simulink model "Simulatore_COMAU_UBHAND_IV_manipolazione_interna"  (Simulink 7.6)
settings: Solver=ode45  StartTime=0.0  StopTime=inf  RelTol=1e-3
values from neighbouring files (symbols this model uses):
  a_f = zeros(4,5)    [KinematicData.m]
  alpha_f = zeros(4,5)    [KinematicData.m]
  d_f = zeros(4,5)    [KinematicData.m]
  dc4_1 = 660    [KinematicData.m]
  T6HAND = [1 0 0 0;0 1 0 0; 0 0 1 357.48+95; 0 0 0 1]    [KinematicData.m]
  teta_d = atan(647.07/130)    [KinematicData.m]
  teta_f = zeros(4,5)    [KinematicData.m]
undefined symbols (not defined in the file or in its folder): a_c, a_w, alpha_c, alpha_w, d_c, d_w, Dt_fingers, teta_c
libraries: simulink x281, dspmtrx3 x171, aerolibutil x163, aerolibconvert2 x64, simulink_extras x46, vrlib x26, aerolibtransform2 x7, dspnetwork x1
(part 3 of 15)

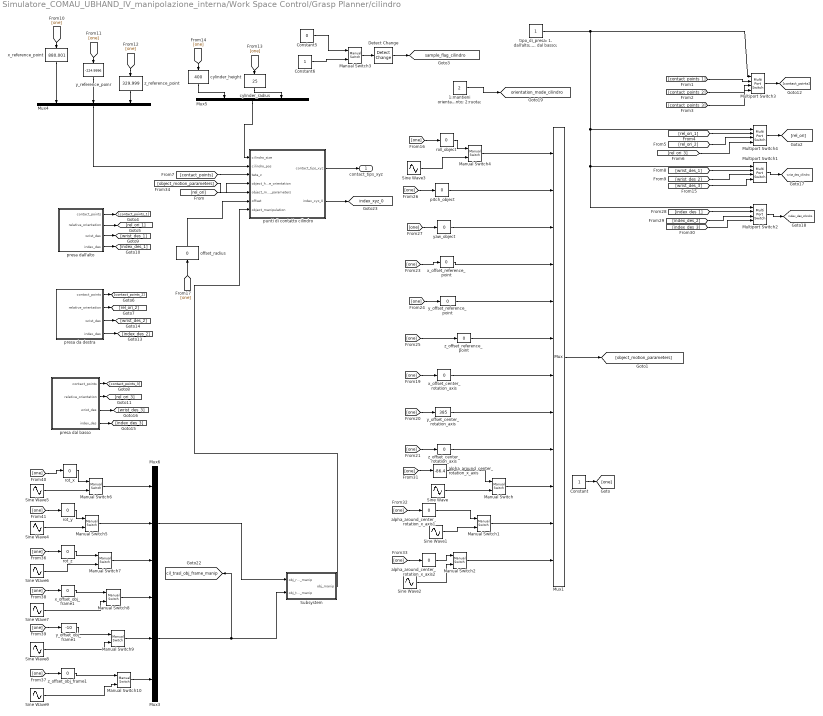
== system Simulatore_COMAU_UBHAND_IV_manipolazione_interna/Work Space Control/Grasp Planner/cilindro ==
  1:mantieni orientamento; 2:ruota; : Constant  Value=2
  Constant : Constant
  Constant5 : Constant  Value=0
  Constant6 : Constant
  Detect Change : simulink/Logic and Bit Operations/Detect Change  vinit=0  InputProcessing=Inherited  OutDataTypeStr=uint8
  Manual Switch{,1..3,5..10} (10 alike) : simulink/Signal Routing/Manual Switch  sw=1  action=0  varsize=off
  Manual Switch4 : simulink/Signal Routing/Manual Switch  sw=0  action=0  varsize=off
  Multiport Switch{1..4} (4 alike) : MultiPortSwitch  zeroidx=off
  Mux1 : Mux  Inputs=12
  Mux3 : Mux  Inputs=3
  Mux4 : Mux  Inputs=3
  Mux5 : Mux  Inputs=2
  Mux6 : Mux  Inputs=3
  Sine Wave : Sin  Amplitude=60  SampleTime=0.01
  Sine Wave1 : Sin  Amplitude=30  SampleTime=0.01
  Sine Wave2 : Sin  Amplitude=90  Bias=90+180  SampleTime=0.01
  Sine Wave3 : Sin  Amplitude=30  Phase=pi  SampleTime=0.01
  Sine Wave4 : Sin  Amplitude=10  Phase=pi/2  SampleTime=0.01
  Sine Wave5 : Sin  Amplitude=10  SampleTime=0.01
  Sine Wave6 : Sin  Amplitude=10  Bias=-5  SampleTime=0.01
  Sine Wave7 : Sin  Amplitude=10  Bias=-5  SampleTime=0.01
  Sine Wave8 : Sin  Amplitude=10  Bias=-20  Phase=pi/2  SampleTime=0.01
  Sine Wave9 : Sin  Amplitude=10  Bias=-5  SampleTime=0.01
  Subsystem : Subsystem
  alpha_around_center_rotation_x_axis : simulink/Math Operations/Slider Gain  low=-180  gain=-86.4  high=180
  alpha_around_center_rotation_x_axis1 : simulink/Math Operations/Slider Gain  low=-180  gain=0  high=180
  alpha_around_center_rotation_x_axis2 : simulink/Math Operations/Slider Gain  low=-180  gain=0  high=180
  cylinder_height : simulink/Math Operations/Slider Gain  low=0  gain=400  high=500
  cylinder_radius : simulink/Math Operations/Slider Gain  low=0  gain=25  high=500
  offset_radius : simulink/Math Operations/Slider Gain  low=0  gain=0  high=500
  pitch_object : simulink/Math Operations/Slider Gain  low=-180  gain=0  high=180
  presa da destra : Subsystem
  presa dal basso : Subsystem
  presa dall'alto : Subsystem
  punti di contatto cilindro : Subsystem
  roll_object : simulink/Math Operations/Slider Gain  low=-180  gain=0  high=180
  rot_x : simulink/Math Operations/Slider Gain  low=-180  gain=0  high=180
  rot_y : simulink/Math Operations/Slider Gain  low=-180  gain=0  high=180
  rot_z : simulink/Math Operations/Slider Gain  low=-20  gain=0  high=20
  tipo_di_presa: 1. dall'alto; 2. da destra; 3. dal basso; : Constant
  x_offset_center_rotation_axis : simulink/Math Operations/Slider Gain  low=0  gain=0  high=500
  x_offset_obj_frame1 : simulink/Math Operations/Slider Gain  low=-50  gain=0  high=50
  x_offset_reference_point : simulink/Math Operations/Slider Gain  low=0  gain=0  high=500
  x_reference_point : simulink/Math Operations/Slider Gain  low=-1000  gain=880.001  high=2000
  y_offset_center_rotation_axis : simulink/Math Operations/Slider Gain  low=0  gain=385  high=500
  y_offset_obj_frame1 : simulink/Math Operations/Slider Gain  low=-40  gain=-10  high=40
  y_offset_reference_point : simulink/Math Operations/Slider Gain  low=0  gain=0  high=500
  y_reference_poinr : simulink/Math Operations/Slider Gain  low=-1500  gain=-224.9996  high=800
  yaw_object : simulink/Math Operations/Slider Gain  low=-180  gain=0  high=180
  z_offset_center_rotation_axis : simulink/Math Operations/Slider Gain  low=-1000  gain=0  high=500
  z_offset_obj_frame1 : simulink/Math Operations/Slider Gain  low=-50  gain=0  high=50
  z_offset_reference_point : simulink/Math Operations/Slider Gain  low=-1000  gain=0  high=500
  z_reference_point : simulink/Math Operations/Slider Gain  low=-1000  gain=329.999  high=2000
  contact_tips_xyz : Outport
  tag scope: cil_trasl_obj_frame_manip=global, index_des_cilindro=global, index_xyz_0=global, orientation_mode_cilindro=global, sample_flag_cilindro=global, wrist_des_cilindro=global
  wires:
  [one] -> x_reference_point/1
  x_reference_point/1 -> Mux4/1
  [one] -> y_reference_poinr/1
  y_reference_poinr/1 -> Mux4/2
  [one] -> z_reference_point/1
  z_reference_point/1 -> Mux4/3
  Mux4/1 -> punti di contatto cilindro/2(cilindro_pos)
  [one] -> cylinder_radius/1
  [one] -> cylinder_height/1
  Mux5/1 -> punti di contatto cilindro/1(cilindro_size)
  cylinder_height/1 -> Mux5/1
  cylinder_radius/1 -> Mux5/2
  punti di contatto cilindro/1(contact_tips_xyz) -> contact_tips_xyz/1
  [one] -> roll_object/1
  [one] -> pitch_object/1
  [one] -> yaw_object/1
  [one] -> alpha_around_center_rotation_x_axis/1
  [one] -> x_offset_center_rotation_axis/1
  [one] -> y_offset_center_rotation_axis/1
  [one] -> z_offset_center_rotation_axis/1
  [one] -> z_offset_reference_point/1
  [one] -> y_offset_reference_point/1
  [one] -> x_offset_reference_point/1
  Mux1/1 -> [object_motion_parameters]
  [rel_ori] -> punti di contatto cilindro/4(object_hand_relative_orientation)
  [object_motion_parameters] -> punti di contatto cilindro/5(object_motion_parameters)
  Constant/1 -> [one]
  tipo_di_presa: 1. dall'alto; 2. da destra; 3. dal basso;/1 -> Multiport Switch3/1, Multiport Switch4/1, Multiport Switch1/1, Multiport Switch2/1
  presa dall'alto/1(contact_points) -> [contact_points_1]
  presa dall'alto/2(relative_orientation) -> [rel_ori_1]
  presa da destra/1(contact_points) -> [contact_points_2]
  presa da destra/2(relative_orientation) -> [rel_ori_2]
  presa dal basso/1(contact_points) -> [contact_points_3]
  presa dal basso/2(relative_orientation) -> [rel_ori_3]
  [rel_ori_3] -> Multiport Switch4/4
  [rel_ori_2] -> Multiport Switch4/3
  [rel_ori_1] -> Multiport Switch4/2
  [contact_points_1] -> Multiport Switch3/2
  [contact_points_2] -> Multiport Switch3/3
  [contact_points_3] -> Multiport Switch3/4
  Multiport Switch3/1 -> [contact_points]
  [contact_points] -> punti di contatto cilindro/3(teta_z)
  Multiport Switch4/1 -> [rel_ori]
  presa dall'alto/3(wrist_des) -> [wrist_des_1]
  presa dall'alto/4(index_des) -> [index_des_1]
  presa da destra/3(wrist_des) -> [wrist_des_2]
  presa da destra/4(index_des) -> [index_des_2]
  presa dal basso/3(wrist_des) -> [wrist_des_3]
  presa dal basso/4(index_des) -> [index_des_3]
  [wrist_des_1] -> Multiport Switch1/2
  [wrist_des_2] -> Multiport Switch1/3
  [wrist_des_3] -> Multiport Switch1/4
  [index_des_1] -> Multiport Switch2/2
  [index_des_3] -> Multiport Switch2/4
  [index_des_2] -> Multiport Switch2/3
  Multiport Switch1/1 -> [wrist_des_cilindro]
  Multiport Switch2/1 -> [index_des_cilindro]
  1:mantieni orientamento; 2:ruota;/1 -> [orientation_mode_cilindro]
  pitch_object/1 -> Mux1/2
  yaw_object/1 -> Mux1/3
  x_offset_reference_point/1 -> Mux1/4
  y_offset_reference_point/1 -> Mux1/5
  z_offset_reference_point/1 -> Mux1/6
  x_offset_center_rotation_axis/1 -> Mux1/7
  y_offset_center_rotation_axis/1 -> Mux1/8
  z_offset_center_rotation_axis/1 -> Mux1/9
  [one] -> alpha_around_center_rotation_x_axis1/1
  [one] -> alpha_around_center_rotation_x_axis2/1
  alpha_around_center_rotation_x_axis/1 -> Manual Switch/1
  Sine Wave/1 -> Manual Switch/2
  Manual Switch/1 -> Mux1/10
  alpha_around_center_rotation_x_axis1/1 -> Manual Switch1/1
  Sine Wave1/1 -> Manual Switch1/2
  Manual Switch1/1 -> Mux1/11
  alpha_around_center_rotation_x_axis2/1 -> Manual Switch2/1
  Sine Wave2/1 -> Manual Switch2/2
  Manual Switch2/1 -> Mux1/12
  Constant6/1 -> Manual Switch3/2
  Constant5/1 -> Manual Switch3/1
  Manual Switch3/1 -> Detect Change/1
  Detect Change/1 -> [sample_flag_cilindro]
  [one] -> offset_radius/1
  offset_radius/1 -> punti di contatto cilindro/6(offset)
  Sine Wave3/1 -> Manual Switch4/2
  roll_object/1 -> Manual Switch4/1
  Manual Switch4/1 -> Mux1/1
  [one] -> z_offset_obj_frame1/1
  [one] -> y_offset_obj_frame1/1
  [one] -> x_offset_obj_frame1/1
  [one] -> rot_z/1
  [one] -> rot_y/1
  [one] -> rot_x/1
  Mux3/1 -> Subsystem/2(obj_trasl_manip), [cil_trasl_obj_frame_manip]
  Mux6/1 -> Subsystem/1(obj_rotat_manip)
  punti di contatto cilindro/2(index_xyz_0) -> [index_xyz_0]
  Subsystem/1(obj_manip) -> punti di contatto cilindro/7(object_manipulation)
  Sine Wave4/1 -> Manual Switch5/2
  rot_y/1 -> Manual Switch5/1
  Manual Switch5/1 -> Mux6/2
  Sine Wave5/1 -> Manual Switch6/2
  rot_x/1 -> Manual Switch6/1
  Manual Switch6/1 -> Mux6/1
  Sine Wave6/1 -> Manual Switch7/2
  Manual Switch7/1 -> Mux6/3
  rot_z/1 -> Manual Switch7/1
  Manual Switch8/1 -> Mux3/1
  x_offset_obj_frame1/1 -> Manual Switch8/1
  Sine Wave7/1 -> Manual Switch8/2
  Sine Wave8/1 -> Manual Switch9/2
  y_offset_obj_frame1/1 -> Manual Switch9/1
  Manual Switch9/1 -> Mux3/2
  Sine Wave9/1 -> Manual Switch10/2
  z_offset_obj_frame1/1 -> Manual Switch10/1
  Manual Switch10/1 -> Mux3/3

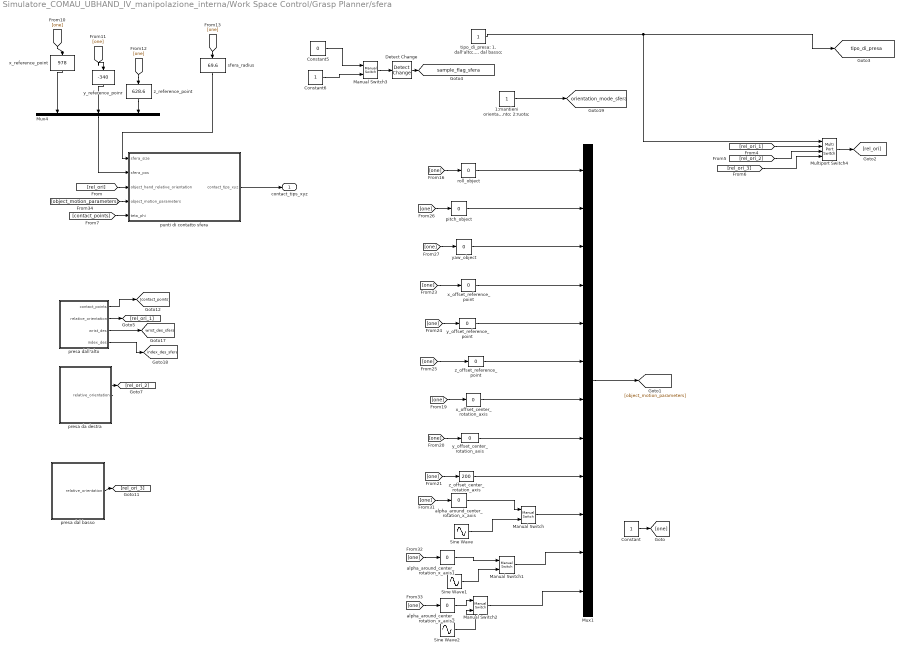
== system Simulatore_COMAU_UBHAND_IV_manipolazione_interna/Work Space Control/Grasp Planner/sfera ==
  1:mantieni orientamento; 2:ruota; : Constant
  Constant : Constant
  Constant5 : Constant  Value=0
  Constant6 : Constant
  Detect Change : simulink/Logic and Bit Operations/Detect Change  vinit=0  InputProcessing=Inherited  OutDataTypeStr=uint8
  Manual Switch{,1..3} (4 alike) : simulink/Signal Routing/Manual Switch  sw=1  action=0  varsize=off
  Multiport Switch4 : MultiPortSwitch  zeroidx=off
  Mux1 : Mux  Inputs=12
  Mux4 : Mux  Inputs=3
  Sine Wave : Sin  Amplitude=60  Bias=30  SampleTime=0
  Sine Wave1 : Sin  Amplitude=60  Bias=30  SampleTime=0
  Sine Wave2 : Sin  Amplitude=3600  Frequency=0.1  Phase=pi  SampleTime=0
  alpha_around_center_rotation_x_axis : simulink/Math Operations/Slider Gain  low=-180  gain=0  high=180
  alpha_around_center_rotation_x_axis1 : simulink/Math Operations/Slider Gain  low=-180  gain=0  high=180
  alpha_around_center_rotation_x_axis2 : simulink/Math Operations/Slider Gain  low=-180  gain=0  high=180
  pitch_object : simulink/Math Operations/Slider Gain  low=-180  gain=0  high=180
  presa da destra : Subsystem
  presa dal basso : Subsystem
  presa dall'alto : Subsystem
  punti di contatto sfera : Subsystem
  roll_object : simulink/Math Operations/Slider Gain  low=-180  gain=0  high=180
  sfera_radius : simulink/Math Operations/Slider Gain  low=0  gain=69.6  high=90
  tipo_di_presa: 1. dall'alto; 2. da destra; 3. dal basso; : Constant
  x_offset_center_rotation_axis : simulink/Math Operations/Slider Gain  low=0  gain=0  high=500
  x_offset_reference_point : simulink/Math Operations/Slider Gain  low=0  gain=0  high=500
  x_reference_point : simulink/Math Operations/Slider Gain  low=-1000  gain=978  high=2000
  y_offset_center_rotation_axis : simulink/Math Operations/Slider Gain  low=0  gain=0  high=500
  y_offset_reference_point : simulink/Math Operations/Slider Gain  low=0  gain=0  high=500
  y_reference_poinr : simulink/Math Operations/Slider Gain  low=-1000  gain=-340  high=1000
  yaw_object : simulink/Math Operations/Slider Gain  low=-180  gain=0  high=180
  z_offset_center_rotation_axis : simulink/Math Operations/Slider Gain  low=-1000  gain=200  high=500
  z_offset_reference_point : simulink/Math Operations/Slider Gain  low=-1000  gain=0  high=500
  z_reference_point : simulink/Math Operations/Slider Gain  low=-1000  gain=628.6  high=1370
  contact_tips_xyz : Outport
  tag scope: index_des_sfera=global, orientation_mode_sfera=global, sample_flag_sfera=global, tipo_di_presa=global, wrist_des_sfera=global
  wires:
  1:mantieni orientamento; 2:ruota;/1 -> [orientation_mode_sfera]
  Multiport Switch4/1 -> [rel_ori]
  [rel_ori_1] -> Multiport Switch4/2
  [rel_ori_2] -> Multiport Switch4/3
  [rel_ori_3] -> Multiport Switch4/4
  presa dal basso/1(relative_orientation) -> [rel_ori_3]
  presa da destra/1(relative_orientation) -> [rel_ori_2]
  presa dall'alto/2(relative_orientation) -> [rel_ori_1]
  tipo_di_presa: 1. dall'alto; 2. da destra; 3. dal basso;/1 -> Multiport Switch4/1, [tipo_di_presa]
  Constant/1 -> [one]
  Mux1/1 -> [object_motion_parameters]
  z_reference_point/1 -> Mux4/3
  [one] -> z_reference_point/1
  y_reference_poinr/1 -> Mux4/2
  [one] -> y_reference_poinr/1
  x_reference_point/1 -> Mux4/1
  [one] -> x_reference_point/1
  [one] -> sfera_radius/1
  sfera_radius/1 -> punti di contatto sfera/1(sfera_size)
  Mux4/1 -> punti di contatto sfera/2(sfera_pos)
  [contact_points] -> punti di contatto sfera/5(teta_phi)
  [rel_ori] -> punti di contatto sfera/3(object_hand_relative_orientation)
  [object_motion_parameters] -> punti di contatto sfera/4(object_motion_parameters)
  punti di contatto sfera/1(contact_tips_xyz) -> contact_tips_xyz/1
  [one] -> roll_object/1
  [one] -> pitch_object/1
  [one] -> yaw_object/1
  [one] -> alpha_around_center_rotation_x_axis/1
  [one] -> x_offset_center_rotation_axis/1
  [one] -> y_offset_center_rotation_axis/1
  [one] -> z_offset_center_rotation_axis/1
  [one] -> z_offset_reference_point/1
  [one] -> y_offset_reference_point/1
  [one] -> x_offset_reference_point/1
  roll_object/1 -> Mux1/1
  pitch_object/1 -> Mux1/2
  yaw_object/1 -> Mux1/3
  x_offset_reference_point/1 -> Mux1/4
  y_offset_reference_point/1 -> Mux1/5
  z_offset_reference_point/1 -> Mux1/6
  x_offset_center_rotation_axis/1 -> Mux1/7
  y_offset_center_rotation_axis/1 -> Mux1/8
  z_offset_center_rotation_axis/1 -> Mux1/9
  [one] -> alpha_around_center_rotation_x_axis1/1
  [one] -> alpha_around_center_rotation_x_axis2/1
  alpha_around_center_rotation_x_axis/1 -> Manual Switch/1
  Sine Wave/1 -> Manual Switch/2
  Manual Switch/1 -> Mux1/10
  alpha_around_center_rotation_x_axis1/1 -> Manual Switch1/1
  Sine Wave1/1 -> Manual Switch1/2
  Manual Switch1/1 -> Mux1/11
  alpha_around_center_rotation_x_axis2/1 -> Manual Switch2/1
  Sine Wave2/1 -> Manual Switch2/2
  Manual Switch2/1 -> Mux1/12
  presa dall'alto/3(wrist_des) -> [wrist_des_sfera]
  presa dall'alto/4(index_des) -> [index_des_sfera]
  presa dall'alto/1(contact_points) -> [contact_points]
  Constant6/1 -> Manual Switch3/2
  Constant5/1 -> Manual Switch3/1
  Manual Switch3/1 -> Detect Change/1
  Detect Change/1 -> [sample_flag_sfera]

== system Simulatore_COMAU_UBHAND_IV_manipolazione_interna/Work Space Control/Inverse_kinematic/Inverse_kinematic_comau ==
  end_effector_xyz : Inport
  wrist_angles_des : Inport  Port=2
  index_angles_des : Inport  Port=3
  Angle Conversion : aerolibconvert2/Angle Conversion  IU=rad  OU=deg
  Constant : Constant
  Constant1 : Constant  Value=2
  Display : Display
  Gain : Gain  Gain=-1
  Manual Switch : simulink/Signal Routing/Manual Switch  sw=1  action=0  varsize=off
  Multiport Switch : MultiPortSwitch  zeroidx=off
  Multiport Switch1 : MultiPortSwitch  zeroidx=off
  Multiport Switch2 : MultiPortSwitch  Inputs=2  zeroidx=off
  Mux1 : Mux  Inputs=2
  R_0index : Subsystem
  Reshape1 : Reshape
  Subsystem : Subsystem
  Subsystem1 : Subsystem
  T6U : Constant  Value=[1 0 0 0;0 1 0 0;0 0 1 0;0 0 0 1]
  T_0_3 : Subsystem
  calcR06,P06 : Subsystem
  calcR06,P1 : Subsystem
  first solution : Subsystem
  q[1..6] : Outport
  T_0H : Outport  Port=2
  tag scope: T_0_index=global, T_0_object=global
  wires:
  R_0index/1(T_06) -> calcR06,P06/1(4x4 end effector reference)
  T6U/1 -> calcR06,P06/2(T6U)
  calcR06,P06/2(P06) -> Display/1, first solution/1(P06)
  T_0_3/1(T_0_3) -> calcR06,P1/2(R03)
  first solution/1(q[1..3]) -> T_0_3/1(q[1..3]), Mux1/1
  calcR06,P06/1(R06) -> calcR06,P1/1(R06)
  Mux1/1 -> Angle Conversion/1
  Constant/1 -> Manual Switch/1
  Constant1/1 -> Manual Switch/2
  Manual Switch/1 -> Subsystem/2(In1)
  calcR06,P1/1(R36) -> Subsystem/1(R36)
  Subsystem/1(q[4..6]) -> Gain/1
  wrist_angles_des/1 -> R_0index/1(wrist_angles_des1)
  index_angles_des/1 -> R_0index/2(index_angles_des1)
  end_effector_xyz/1 -> Subsystem1/1(end_effector_xyz)
  Subsystem1/1(T_0_end_effector) -> R_0index/3(T_0_index), [T_0_index]
  Angle Conversion/1 -> q[1..6]/1
  R_0index/2(T_0H) -> T_0H/1
  [T_0_object_tip_sfera] -> Multiport Switch/2
  [T_0_object_tip_box] -> Multiport Switch/3
  [T_0_object_tip_cilindro] -> Multiport Switch/4
  [current_object] -> Multiport Switch/1
  Multiport Switch/1 -> R_0index/4(T_0_object), Reshape1/1
  [R_0_end_effector_sfera] -> Multiport Switch1/2
  [R_0_end_effector_box] -> Multiport Switch1/3
  [R_0_end_effector_cilindro] -> Multiport Switch1/4
  [current_object] -> Multiport Switch1/1
  Multiport Switch1/1 -> Multiport Switch2/3
  [R_end_effector_index] -> Multiport Switch2/2
  [reference_mode] -> Multiport Switch2/1
  Multiport Switch2/1 -> Subsystem1/2(R_0_end_effector)
  Reshape1/1 -> [T_0_object]
  Gain/1 -> Mux1/2

== system Simulatore_COMAU_UBHAND_IV_manipolazione_interna/Work Space Control/Inverse_kinematic/Inverse_kinematic_hand ==
  xyz_thumb : Inport
  xyz_index : Inport  Port=2
  xyz_middle : Inport  Port=3
  xyz_ring : Inport  Port=4
  xyz_little : Inport  Port=5
  Inverse_kinematic_little : Subsystem
  Inverse_kinematic_middle : Subsystem
  Inverse_kinematic_middle1 : Subsystem
  Inverse_kinematic_ring : Subsystem
  Inverse_kinematic_thumb : Subsystem
  Submatrix{,1..4} (5 alike) : dspmtrx3/Submatrix  RowSpan=Range of rows  RowStartMode=Index  RowStartIndex=1  RowEndMode=Index  RowEndIndex=3  ColSpan=One column  ColStartMode=Index  ColStartIndex=1  ColEndMode=Index  ColEndIndex=3
  teta[1..4]{,1..4} (5 alike) : Display
  xyz{1,5,7..9} (5 alike) : dspmtrx3/Submatrix  RowSpan=Range of rows  RowStartMode=Index  RowStartIndex=1  RowEndMode=Index  RowEndIndex=3  ColSpan=One column  ColStartMode=Index  ColStartIndex=1  ColEndMode=Index  ColEndIndex=3
  Thumb : Outport
  Index : Outport  Port=2
  Middle : Outport  Port=3
  Ring : Outport  Port=4
  Little : Outport  Port=5
  wires:
  Inverse_kinematic_thumb/1(q_thumb) -> teta[1..4]/1, xyz5/1
  Inverse_kinematic_little/1(q_index) -> teta[1..4]4/1, xyz9/1
  Inverse_kinematic_ring/1(q_index) -> teta[1..4]3/1, xyz8/1
  Inverse_kinematic_middle/1(q_index) -> teta[1..4]2/1, xyz7/1
  xyz5/1 -> Thumb/1
  xyz7/1 -> Middle/1
  xyz8/1 -> Ring/1
  xyz9/1 -> Little/1
  xyz_thumb/1 -> Submatrix/1
  xyz_middle/1 -> Submatrix1/1
  xyz_ring/1 -> Submatrix2/1
  xyz_little/1 -> Submatrix3/1
  Submatrix/1 -> Inverse_kinematic_thumb/1(xyz_finger_base_tip)
  Submatrix1/1 -> Inverse_kinematic_middle/1(xyz_finger_base_tip)
  Submatrix2/1 -> Inverse_kinematic_ring/1(xyz_finger_base_tip)
  Submatrix3/1 -> Inverse_kinematic_little/1(xyz_finger_base_tip)
  Inverse_kinematic_middle1/1(q_index) -> xyz1/1
  xyz_index/1 -> Submatrix4/1
  Submatrix4/1 -> Inverse_kinematic_middle1/1(xyz_finger_base_tip)
  xyz1/1 -> Index/1, teta[1..4]1/1

== system Simulatore_COMAU_UBHAND_IV_manipolazione_interna/Work Space Control/Inverse_kinematic/Tf0 ==
  T_0HAND : Inport
  wrist_angles[1..2] : Inport  Port=2
  fingers_references_xyz 0 : Inport  Port=3
  Constant : Constant  Value=Dt_fingers(1).T_w2_finger_base  VectorParams1D=off
  Constant1 : Constant  Value=Dt_fingers(2).T_w2_finger_base  VectorParams1D=off
  Constant4 : Constant  Value=Dt_fingers(3).T_w2_finger_base  VectorParams1D=off
  Constant5 : Constant  Value=Dt_fingers(4).T_w2_finger_base  VectorParams1D=off
  Constant6 : Constant  Value=Dt_fingers(5).T_w2_finger_base  VectorParams1D=off
  Demux : Demux  Outputs=5
  Demux3 : Demux  Outputs=2
  Matrix Multiply{1..10} (10 alike) : Product  Multiplication=Matrix(*)
  Mux : Mux  Inputs=2
  Mux1 : Mux  Inputs=5
  T^-1 : Subsystem
  T^-2 : Subsystem
  T^-3 : Subsystem
  T^-4 : Subsystem
  T^-5 : Subsystem
  T_0_8 : Subsystem
  teta[1..4] : Display
  fingers_references xyz fingers_references 1 : Outport
  wires:
  T_0_8/1(T_08) -> Matrix Multiply1/1, Matrix Multiply3/1, Matrix Multiply4/1, Matrix Multiply5/1, Matrix Multiply2/1
  wrist_angles[1..2]/1 -> Demux3/1
  Mux/1 -> T_0_8/1(wrist_angles[1..2])
  Demux3/2 -> Mux/2
  Demux3/1 -> Mux/1
  T_0HAND/1 -> T_0_8/2(T_0H)
  Constant6/1 -> Matrix Multiply5/2
  Constant5/1 -> Matrix Multiply4/2
  Constant4/1 -> Matrix Multiply3/2
  Constant/1 -> Matrix Multiply1/2
  Matrix Multiply1/1 -> T^-1/1(T)
  Matrix Multiply3/1 -> T^-3/1(T)
  Matrix Multiply4/1 -> T^-4/1(T)
  Matrix Multiply5/1 -> T^-5/1(T)
  T^-3/1(T^-1) -> Matrix Multiply8/1
  T^-4/1(T^-1) -> Matrix Multiply9/1
  T^-5/1(T^-1) -> Matrix Multiply10/1
  T^-1/1(T^-1) -> Matrix Multiply6/1
  Mux1/1 -> fingers_references xyz fingers_references 1/1, teta[1..4]/1
  Matrix Multiply10/1 -> Mux1/5
  Matrix Multiply9/1 -> Mux1/4
  Matrix Multiply8/1 -> Mux1/3
  Matrix Multiply6/1 -> Mux1/1
  Matrix Multiply7/1 -> Mux1/2
  T^-2/1(T^-1) -> Matrix Multiply7/1
  Matrix Multiply2/1 -> T^-2/1(T)
  Constant1/1 -> Matrix Multiply2/2
  fingers_references_xyz 0/1 -> Demux/1
  Demux/5 -> Matrix Multiply10/2
  Demux/4 -> Matrix Multiply9/2
  Demux/3 -> Matrix Multiply8/2
  Demux/2 -> Matrix Multiply7/2
  Demux/1 -> Matrix Multiply6/2

== system Simulatore_COMAU_UBHAND_IV_manipolazione_interna/Work Space Control/R_end_effector/rot_x ==
  alpha : Inport
  Angle Conversion : aerolibconvert2/Angle Conversion  IU=deg  OU=rad
  Constant{,1..2,5} (4 alike) : Constant  Value=0
  Constant4 : Constant
  Create 3x3 Matrix : aerolibutil/Create 3x3 Matrix
  Gain : Gain  Gain=-1
  Trigonometric Function : Trigonometry
  Trigonometric Function1 : Trigonometry  Operator=cos
  Trigonometric Function2 : Trigonometry
  Trigonometric Function3 : Trigonometry  Operator=cos
  rotx : Outport
  wires:
  alpha/1 -> Angle Conversion/1
  Angle Conversion/1 -> Trigonometric Function3/1, Trigonometric Function2/1, Trigonometric Function/1, Trigonometric Function1/1
  Create 3x3 Matrix/1 -> rotx/1
  Constant4/1 -> Create 3x3 Matrix/1
  Constant2/1 -> Create 3x3 Matrix/2
  Constant/1 -> Create 3x3 Matrix/3
  Constant5/1 -> Create 3x3 Matrix/4
  Trigonometric Function3/1 -> Create 3x3 Matrix/5
  Trigonometric Function2/1 -> Gain/1
  Gain/1 -> Create 3x3 Matrix/6
  Constant1/1 -> Create 3x3 Matrix/7
  Trigonometric Function/1 -> Create 3x3 Matrix/8
  Trigonometric Function1/1 -> Create 3x3 Matrix/9

== system Simulatore_COMAU_UBHAND_IV_manipolazione_interna/Work Space Control/R_end_effector/rot_y ==
  beta : Inport
  Angle Conversion : aerolibconvert2/Angle Conversion  IU=deg  OU=rad
  Constant{1..3,5} (4 alike) : Constant  Value=0
  Constant4 : Constant
  Create 3x3 Matrix : aerolibutil/Create 3x3 Matrix
  Gain : Gain  Gain=-1
  Trigonometric Function : Trigonometry
  Trigonometric Function1 : Trigonometry  Operator=cos
  Trigonometric Function4 : Trigonometry  Operator=cos
  Trigonometric Function5 : Trigonometry
  roty : Outport
  wires:
  Constant1/1 -> Create 3x3 Matrix/8
  Gain/1 -> Create 3x3 Matrix/7
  Trigonometric Function4/1 -> Create 3x3 Matrix/1
  Trigonometric Function1/1 -> Create 3x3 Matrix/9
  Trigonometric Function/1 -> Gain/1
  Constant3/1 -> Create 3x3 Matrix/6
  Constant4/1 -> Create 3x3 Matrix/5
  Constant5/1 -> Create 3x3 Matrix/4
  Trigonometric Function5/1 -> Create 3x3 Matrix/3
  Constant2/1 -> Create 3x3 Matrix/2
  Create 3x3 Matrix/1 -> roty/1
  Angle Conversion/1 -> Trigonometric Function4/1, Trigonometric Function5/1, Trigonometric Function/1, Trigonometric Function1/1
  beta/1 -> Angle Conversion/1

== system Simulatore_COMAU_UBHAND_IV_manipolazione_interna/Work Space Control/R_end_effector/rot_z ==
  gamma : Inport
  Angle Conversion : aerolibconvert2/Angle Conversion  IU=deg  OU=rad
  Constant{,1..3} (4 alike) : Constant  Value=0
  Constant4 : Constant
  Create 3x3 Matrix : aerolibutil/Create 3x3 Matrix
  Gain1 : Gain  Gain=-1
  Trigonometric Function3 : Trigonometry  Operator=cos
  Trigonometric Function4 : Trigonometry  Operator=cos
  Trigonometric Function5 : Trigonometry
  Trigonometric Function6 : Trigonometry
  rotz : Outport
  wires:
  Trigonometric Function5/1 -> Gain1/1
  Constant4/1 -> Create 3x3 Matrix/9
  Constant3/1 -> Create 3x3 Matrix/8
  Constant1/1 -> Create 3x3 Matrix/7
  Constant2/1 -> Create 3x3 Matrix/6
  Trigonometric Function3/1 -> Create 3x3 Matrix/5
  Trigonometric Function6/1 -> Create 3x3 Matrix/4
  Constant/1 -> Create 3x3 Matrix/3
  Gain1/1 -> Create 3x3 Matrix/2
  Trigonometric Function4/1 -> Create 3x3 Matrix/1
  Create 3x3 Matrix/1 -> rotz/1
  Angle Conversion/1 -> Trigonometric Function4/1, Trigonometric Function5/1, Trigonometric Function6/1, Trigonometric Function3/1
  gamma/1 -> Angle Conversion/1

== system Simulatore_COMAU_UBHAND_IV_manipolazione_interna/Joint Space Control/Direct_kinematic2/Direct_kinematic_Comau/T_0_3 ==
  q[1..3] : Inport
  Add : Sum
  Add1 : Sum
  Add2 : Sum  Inputs=-+
  Constant : Constant  Value=[alpha_c(1) alpha_c(2) alpha_c(3) ]
  Constant1 : Constant  Value=teta_c(2)
  Constant2 : Constant  Value=[a_c(1) a_c(2) a_c(3)]
  Constant3 : Constant  Value=teta_c(3)
  Constant4 : Constant  Value=teta_c(1)
  Degrees to Radians : simulink_extras/Transformations/Degrees to Radians
  Demux : Demux  Outputs=3
  Demux1 : Demux  Outputs=3
  Demux2 : Demux  Outputs=3
  Display3 : Display
  Matrix Multiply : Product  Multiplication=Matrix(*)
  Matrix Multiply1 : Product  Multiplication=Matrix(*)
  Subsystem : Subsystem
  Subsystem1 : Subsystem
  Subsystem2 : Subsystem
  T_0_3 : Outport
  wires:
  Constant/1 -> Demux1/1
  Subsystem/1(Out1) -> Matrix Multiply/1
  Subsystem1/1(Out1) -> Matrix Multiply/2
  Matrix Multiply/1 -> Matrix Multiply1/1
  Subsystem2/1(Out1) -> Matrix Multiply1/2
  Matrix Multiply1/1 -> T_0_3/1
  Constant2/1 -> Demux2/1
  Demux2/1 -> Subsystem/3(a)
  Demux2/2 -> Subsystem1/3(a)
  Demux2/3 -> Subsystem2/3(a)
  Demux1/1 -> Subsystem/2(alpha)
  Demux1/2 -> Subsystem1/2(alpha)
  Demux1/3 -> Subsystem2/2(alpha)
  Degrees to Radians/1 -> Demux/1
  q[1..3]/1 -> Degrees to Radians/1, Display3/1
  Add/1 -> Subsystem1/1(q)
  Constant1/1 -> Add/2
  Demux/2 -> Add/1
  Constant3/1 -> Add1/2
  Demux/3 -> Add1/1
  Add1/1 -> Subsystem2/1(q)
  Demux/1 -> Add2/1
  Add2/1 -> Subsystem/1(q)
  Constant4/1 -> Add2/2

== system Simulatore_COMAU_UBHAND_IV_manipolazione_interna/Joint Space Control/Direct_kinematic2/Direct_kinematic_Comau/T_3_6 ==
  q[1..3] : Inport
  Add : Sum  Inputs=-+
  Add1 : Sum  Inputs=-+
  Add2 : Sum  Inputs=-+
  Constant : Constant  Value=[alpha_c(4) alpha_c(5) alpha_c(6) ]
  Constant1 : Constant  Value=teta_c(5)
  Constant2 : Constant  Value=[a_c(4) a_c(5) a_c(6)]
  Constant3 : Constant  Value=teta_c(6)
  Constant4 : Constant  Value=teta_c(4)
  Degrees to Radians : simulink_extras/Transformations/Degrees to Radians
  Demux : Demux  Outputs=3
  Demux1 : Demux  Outputs=3
  Demux2 : Demux  Outputs=3
  Display3 : Display
  Matrix Multiply : Product  Multiplication=Matrix(*)
  Matrix Multiply1 : Product  Multiplication=Matrix(*)
  Subsystem : Subsystem
  Subsystem1 : Subsystem
  Subsystem2 : Subsystem
  T_3_6 : Outport
  wires:
  q[1..3]/1 -> Display3/1, Degrees to Radians/1
  Degrees to Radians/1 -> Demux/1
  Demux1/3 -> Subsystem2/2(alpha)
  Demux1/2 -> Subsystem1/2(alpha)
  Demux1/1 -> Subsystem/2(alpha)
  Demux2/3 -> Subsystem2/3(a)
  Demux2/2 -> Subsystem1/3(a)
  Demux2/1 -> Subsystem/3(a)
  Constant2/1 -> Demux2/1
  Matrix Multiply1/1 -> T_3_6/1
  Subsystem2/1(Out1) -> Matrix Multiply1/2
  Matrix Multiply/1 -> Matrix Multiply1/1
  Subsystem1/1(Out1) -> Matrix Multiply/2
  Subsystem/1(Out1) -> Matrix Multiply/1
  Constant/1 -> Demux1/1
  Add/1 -> Subsystem1/1(q)
  Constant1/1 -> Add/2
  Demux/2 -> Add/1
  Constant3/1 -> Add1/2
  Demux/3 -> Add1/1
  Demux/1 -> Add2/1
  Add2/1 -> Subsystem/1(q)
  Constant4/1 -> Add2/2
  Add1/1 -> Subsystem2/1(q)

== system Simulatore_COMAU_UBHAND_IV_manipolazione_interna/Joint Space Control/Direct_kinematic2/Direct_kinematic_hand/Direct_kinematic_index ==
  Joints_reference : Inport  PortDimensions=3
  T_0f : Inport  Port=2
  Constant : Constant  Value=[alpha_f(2,1) alpha_f(2,2) alpha_f(2,3) alpha_f(2,4)]
  Constant2 : Constant  Value=[a_f(2,1) a_f(2,2) a_f(2,3) a_f(2,4)]
  Degrees to Radians : simulink_extras/Transformations/Degrees to Radians
  Demux : Demux
  Demux1 : Demux
  Demux2 : Demux
  Demux3 : Demux  Outputs=3
  Display3 : Display
  Gain : Gain  Gain=0.7
  Matrix Multiply{,1..3} (4 alike) : Product  Multiplication=Matrix(*)
  Subsystem : Subsystem
  Subsystem1 : Subsystem
  Subsystem2 : Subsystem
  Subsystem3 : Subsystem
  Vector Concatenate : Concatenate  Mode=Vector
  T_finger_tip : Outport
  wires:
  Degrees to Radians/1 -> Demux/1
  Vector Concatenate/1 -> Display3/1, Degrees to Radians/1
  Gain/1 -> Vector Concatenate/2
  Demux3/3 -> Gain/1
  Joints_reference/1 -> Vector Concatenate/1, Demux3/1
  Demux1/4 -> Subsystem3/2(alpha)
  Demux1/3 -> Subsystem2/2(alpha)
  Demux1/2 -> Subsystem1/2(alpha)
  Demux1/1 -> Subsystem/2(alpha)
  Demux2/4 -> Subsystem3/3(a)
  Demux2/3 -> Subsystem2/3(a)
  Demux2/2 -> Subsystem1/3(a)
  Demux2/1 -> Subsystem/3(a)
  Demux/4 -> Subsystem3/1(q)
  Demux/3 -> Subsystem2/1(q)
  Demux/2 -> Subsystem1/1(q)
  Demux/1 -> Subsystem/1(q)
  Constant2/1 -> Demux2/1
  Matrix Multiply2/1 -> T_finger_tip/1
  Matrix Multiply1/1 -> Matrix Multiply2/1
  Subsystem3/1(Out1) -> Matrix Multiply2/2
  Subsystem2/1(Out1) -> Matrix Multiply1/2
  Matrix Multiply/1 -> Matrix Multiply1/1
  Subsystem1/1(Out1) -> Matrix Multiply/2
  Subsystem/1(Out1) -> Matrix Multiply3/2
  Constant/1 -> Demux1/1
  T_0f/1 -> Matrix Multiply3/1
  Matrix Multiply3/1 -> Matrix Multiply/1

== system Simulatore_COMAU_UBHAND_IV_manipolazione_interna/Joint Space Control/Direct_kinematic2/Direct_kinematic_hand/Direct_kinematic_little ==
  Joints_reference : Inport  PortDimensions=3
  T_0f : Inport  Port=2
  Constant : Constant  Value=[alpha_f(5,1) alpha_f(5,2) alpha_f(5,3) alpha_f(5,4)]
  Constant2 : Constant  Value=[a_f(5,1) a_f(5,2) a_f(5,3) a_f(5,4)]
  Degrees to Radians : simulink_extras/Transformations/Degrees to Radians
  Demux : Demux
  Demux1 : Demux
  Demux2 : Demux
  Demux3 : Demux  Outputs=3
  Display3 : Display
  Gain : Gain  Gain=0.7
  Matrix Multiply{,1..3} (4 alike) : Product  Multiplication=Matrix(*)
  Subsystem : Subsystem
  Subsystem1 : Subsystem
  Subsystem2 : Subsystem
  Subsystem3 : Subsystem
  Vector Concatenate : Concatenate  Mode=Vector
  T_finger_tip : Outport
  wires:
  Degrees to Radians/1 -> Demux/1
  Vector Concatenate/1 -> Display3/1, Degrees to Radians/1
  Gain/1 -> Vector Concatenate/2
  Demux3/3 -> Gain/1
  Joints_reference/1 -> Vector Concatenate/1, Demux3/1
  Demux1/4 -> Subsystem3/2(alpha)
  Demux1/3 -> Subsystem2/2(alpha)
  Demux1/2 -> Subsystem1/2(alpha)
  Demux1/1 -> Subsystem/2(alpha)
  Demux2/4 -> Subsystem3/3(a)
  Demux2/3 -> Subsystem2/3(a)
  Demux2/2 -> Subsystem1/3(a)
  Demux2/1 -> Subsystem/3(a)
  Demux/4 -> Subsystem3/1(q)
  Demux/3 -> Subsystem2/1(q)
  Demux/2 -> Subsystem1/1(q)
  Demux/1 -> Subsystem/1(q)
  Constant2/1 -> Demux2/1
  Matrix Multiply2/1 -> T_finger_tip/1
  Matrix Multiply1/1 -> Matrix Multiply2/1
  Subsystem3/1(Out1) -> Matrix Multiply2/2
  Subsystem2/1(Out1) -> Matrix Multiply1/2
  Matrix Multiply/1 -> Matrix Multiply1/1
  Subsystem1/1(Out1) -> Matrix Multiply/2
  Subsystem/1(Out1) -> Matrix Multiply3/2
  Constant/1 -> Demux1/1
  T_0f/1 -> Matrix Multiply3/1
  Matrix Multiply3/1 -> Matrix Multiply/1

== system Simulatore_COMAU_UBHAND_IV_manipolazione_interna/Joint Space Control/Direct_kinematic2/Direct_kinematic_hand/Direct_kinematic_middle ==
  Joints_reference : Inport  PortDimensions=3
  T_0f : Inport  Port=2
  Constant : Constant  Value=[alpha_f(3,1) alpha_f(3,2) alpha_f(3,3) alpha_f(3,4)]
  Constant2 : Constant  Value=[a_f(3,1) a_f(3,2) a_f(3,3) a_f(3,4)]
  Degrees to Radians : simulink_extras/Transformations/Degrees to Radians
  Demux : Demux
  Demux1 : Demux
  Demux2 : Demux
  Demux3 : Demux  Outputs=3
  Display3 : Display
  Gain : Gain  Gain=0.7
  Matrix Multiply{,1..3} (4 alike) : Product  Multiplication=Matrix(*)
  Subsystem : Subsystem
  Subsystem1 : Subsystem
  Subsystem2 : Subsystem
  Subsystem3 : Subsystem
  Vector Concatenate : Concatenate  Mode=Vector
  T_finger_tip : Outport
  wires:
  Constant/1 -> Demux1/1
  Subsystem/1(Out1) -> Matrix Multiply3/2
  Subsystem1/1(Out1) -> Matrix Multiply/2
  Matrix Multiply/1 -> Matrix Multiply1/1
  Subsystem2/1(Out1) -> Matrix Multiply1/2
  Subsystem3/1(Out1) -> Matrix Multiply2/2
  Matrix Multiply1/1 -> Matrix Multiply2/1
  Matrix Multiply2/1 -> T_finger_tip/1
  Constant2/1 -> Demux2/1
  Demux/1 -> Subsystem/1(q)
  Demux/2 -> Subsystem1/1(q)
  Demux/3 -> Subsystem2/1(q)
  Demux/4 -> Subsystem3/1(q)
  Demux2/1 -> Subsystem/3(a)
  Demux2/2 -> Subsystem1/3(a)
  Demux2/3 -> Subsystem2/3(a)
  Demux2/4 -> Subsystem3/3(a)
  Demux1/1 -> Subsystem/2(alpha)
  Demux1/2 -> Subsystem1/2(alpha)
  Demux1/3 -> Subsystem2/2(alpha)
  Demux1/4 -> Subsystem3/2(alpha)
  Joints_reference/1 -> Demux3/1, Vector Concatenate/1
  Demux3/3 -> Gain/1
  Gain/1 -> Vector Concatenate/2
  Vector Concatenate/1 -> Degrees to Radians/1, Display3/1
  Degrees to Radians/1 -> Demux/1
  T_0f/1 -> Matrix Multiply3/1
  Matrix Multiply3/1 -> Matrix Multiply/1

== system Simulatore_COMAU_UBHAND_IV_manipolazione_interna/Joint Space Control/Direct_kinematic2/Direct_kinematic_hand/Direct_kinematic_ring ==
  Joints_reference : Inport  PortDimensions=3
  T_0f : Inport  Port=2
  Constant : Constant  Value=[alpha_f(4,1) alpha_f(4,2) alpha_f(4,3) alpha_f(4,4)]
  Constant2 : Constant  Value=[a_f(4,1) a_f(4,2) a_f(4,3) a_f(4,4)]
  Degrees to Radians : simulink_extras/Transformations/Degrees to Radians
  Demux : Demux
  Demux1 : Demux
  Demux2 : Demux
  Demux3 : Demux  Outputs=3
  Display3 : Display
  Gain : Gain  Gain=0.7
  Matrix Multiply{,1..3} (4 alike) : Product  Multiplication=Matrix(*)
  Subsystem : Subsystem
  Subsystem1 : Subsystem
  Subsystem2 : Subsystem
  Subsystem3 : Subsystem
  Vector Concatenate : Concatenate  Mode=Vector
  T_finger_tip : Outport
  wires:
  Degrees to Radians/1 -> Demux/1
  Vector Concatenate/1 -> Display3/1, Degrees to Radians/1
  Gain/1 -> Vector Concatenate/2
  Demux3/3 -> Gain/1
  Joints_reference/1 -> Vector Concatenate/1, Demux3/1
  Demux1/4 -> Subsystem3/2(alpha)
  Demux1/3 -> Subsystem2/2(alpha)
  Demux1/2 -> Subsystem1/2(alpha)
  Demux1/1 -> Subsystem/2(alpha)
  Demux2/4 -> Subsystem3/3(a)
  Demux2/3 -> Subsystem2/3(a)
  Demux2/2 -> Subsystem1/3(a)
  Demux2/1 -> Subsystem/3(a)
  Demux/4 -> Subsystem3/1(q)
  Demux/3 -> Subsystem2/1(q)
  Demux/2 -> Subsystem1/1(q)
  Demux/1 -> Subsystem/1(q)
  Constant2/1 -> Demux2/1
  Matrix Multiply2/1 -> T_finger_tip/1
  Matrix Multiply1/1 -> Matrix Multiply2/1
  Subsystem3/1(Out1) -> Matrix Multiply2/2
  Subsystem2/1(Out1) -> Matrix Multiply1/2
  Matrix Multiply/1 -> Matrix Multiply1/1
  Subsystem1/1(Out1) -> Matrix Multiply/2
  Subsystem/1(Out1) -> Matrix Multiply3/2
  Constant/1 -> Demux1/1
  T_0f/1 -> Matrix Multiply3/1
  Matrix Multiply3/1 -> Matrix Multiply/1

== system Simulatore_COMAU_UBHAND_IV_manipolazione_interna/Joint Space Control/Direct_kinematic2/Direct_kinematic_hand/Direct_kinematic_thumb ==
  Joints_reference : Inport  PortDimensions=3
  T_0f : Inport  Port=2
  Add{,1..4} (5 alike) : Sum
  Constant : Constant  Value=[alpha_f(1,1) alpha_f(1,2) alpha_f(1,3) alpha_f(1,4)]
  Constant1 : Constant  Value=teta_f(1,1)
  Constant2 : Constant  Value=[a_f(1,1) a_f(1,2) a_f(1,3) a_f(1,4)]
  Constant3 : Constant  Value=teta_f(1,2)
  Constant4 : Constant  Value=teta_f(1,3)
  Constant5 : Constant  Value=teta_f(1,4)
  Constant6 : Constant  Value=-20
  Degrees to Radians : simulink_extras/Transformations/Degrees to Radians
  Demux : Demux
  Demux1 : Demux
  Demux2 : Demux
  Demux3 : Demux  Outputs=3
  Display3 : Display
  Gain : Gain  Gain=0.8
  Matrix Multiply{,1..3} (4 alike) : Product  Multiplication=Matrix(*)
  Subsystem : Subsystem
  Subsystem1 : Subsystem
  Subsystem2 : Subsystem
  Subsystem3 : Subsystem
  Vector Concatenate : Concatenate  Mode=Vector
  T_finger_tip : Outport
  wires:
  Constant/1 -> Demux1/1
  Subsystem/1(Out1) -> Matrix Multiply3/2
  Subsystem1/1(Out1) -> Matrix Multiply/2
  Matrix Multiply/1 -> Matrix Multiply1/1
  Subsystem2/1(Out1) -> Matrix Multiply1/2
  Subsystem3/1(Out1) -> Matrix Multiply2/2
  Matrix Multiply1/1 -> Matrix Multiply2/1
  Matrix Multiply2/1 -> T_finger_tip/1
  Constant2/1 -> Demux2/1
  Demux2/1 -> Subsystem/3(a)
  Demux2/2 -> Subsystem1/3(a)
  Demux2/3 -> Subsystem2/3(a)
  Demux2/4 -> Subsystem3/3(a)
  Demux1/1 -> Subsystem/2(alpha)
  Demux1/2 -> Subsystem1/2(alpha)
  Demux1/3 -> Subsystem2/2(alpha)
  Demux1/4 -> Subsystem3/2(alpha)
  Joints_reference/1 -> Demux3/1, Vector Concatenate/1
  Demux3/3 -> Gain/1
  Vector Concatenate/1 -> Degrees to Radians/1, Display3/1
  Degrees to Radians/1 -> Demux/1
  T_0f/1 -> Matrix Multiply3/1
  Matrix Multiply3/1 -> Matrix Multiply/1
  Constant1/1 -> Add/2
  Demux/1 -> Add/1
  Demux/2 -> Add1/1
  Demux/3 -> Add2/1
  Demux/4 -> Add3/1
  Add3/1 -> Subsystem3/1(q)
  Add2/1 -> Subsystem2/1(q)
  Add1/1 -> Subsystem1/1(q)
  Add/1 -> Subsystem/1(q)
  Constant3/1 -> Add1/2
  Constant4/1 -> Add2/2
  Constant5/1 -> Add3/2
  Constant6/1 -> Add4/2
  Gain/1 -> Add4/1
  Add4/1 -> Vector Concatenate/2

== system Simulatore_COMAU_UBHAND_IV_manipolazione_interna/Joint Space Control/Direct_kinematic2/Direct_kinematic_wrist/T_0_8 ==
  wrist_angles[1..2] : Inport
  T_0HAND : Inport  Port=2
  Add : Sum
  Add1 : Sum
  Constant : Constant  Value=[alpha_w(1) alpha_w(2)]
  Constant1 : Constant  Value=0
  Constant2 : Constant  Value=[a_w(1) a_w(2)]
  Constant3 : Constant  Value=0
  Degrees to Radians : simulink_extras/Transformations/Degrees to Radians
  Demux : Demux  Outputs=2
  Demux1 : Demux  Outputs=2
  Demux2 : Demux  Outputs=2
  Display3 : Display
  Matrix Multiply : Product  Multiplication=Matrix(*)
  Matrix Multiply1 : Product  Multiplication=Matrix(*)
  Matrix Multiply2 : Product  Multiplication=Matrix(*)
  Subsystem : Subsystem
  Subsystem1 : Subsystem
  wrist_reference_ : Subsystem
  T_08 : Outport
  wires:
  wrist_angles[1..2]/1 -> Display3/1, Degrees to Radians/1
  Degrees to Radians/1 -> Demux/1
  Demux1/2 -> Subsystem1/2(alpha)
  Demux1/1 -> Subsystem/2(alpha)
  Demux2/2 -> Subsystem1/3(a)
  Demux2/1 -> Subsystem/3(a)
  Constant2/1 -> Demux2/1
  Matrix Multiply/1 -> T_08/1
  Subsystem1/1(Out1) -> Matrix Multiply/2
  Constant/1 -> Demux1/1
  Subsystem/1(Out1) -> Matrix Multiply1/2
  Matrix Multiply1/1 -> Matrix Multiply/1
  Constant1/1 -> Add/2
  Demux/1 -> Add/1
  Add/1 -> Subsystem/1(q)
  Constant3/1 -> Add1/2
  Demux/2 -> Add1/1
  Add1/1 -> Subsystem1/1(q)
  T_0HAND/1 -> Matrix Multiply2/1
  wrist_reference_/1(T0_W) -> Matrix Multiply2/2
  Matrix Multiply2/1 -> Matrix Multiply1/1

== system Simulatore_COMAU_UBHAND_IV_manipolazione_interna/SCOPES/Direct_kinematic2/Direct_kinematic_Comau/T_0_3 ==
  q[1..3] : Inport
  Add : Sum
  Add1 : Sum
  Add2 : Sum  Inputs=-+
  Constant : Constant  Value=[alpha_c(1) alpha_c(2) alpha_c(3) ]
  Constant1 : Constant  Value=teta_c(2)
  Constant2 : Constant  Value=[a_c(1) a_c(2) a_c(3)]
  Constant3 : Constant  Value=teta_c(3)
  Constant4 : Constant  Value=teta_c(1)
  Degrees to Radians : simulink_extras/Transformations/Degrees to Radians
  Demux : Demux  Outputs=3
  Demux1 : Demux  Outputs=3
  Demux2 : Demux  Outputs=3
  Display3 : Display
  Matrix Multiply : Product  Multiplication=Matrix(*)
  Matrix Multiply1 : Product  Multiplication=Matrix(*)
  Subsystem : Subsystem
  Subsystem1 : Subsystem
  Subsystem2 : Subsystem
  T_0_3 : Outport
  wires:
  Constant/1 -> Demux1/1
  Subsystem/1(Out1) -> Matrix Multiply/1
  Subsystem1/1(Out1) -> Matrix Multiply/2
  Matrix Multiply/1 -> Matrix Multiply1/1
  Subsystem2/1(Out1) -> Matrix Multiply1/2
  Matrix Multiply1/1 -> T_0_3/1
  Constant2/1 -> Demux2/1
  Demux2/1 -> Subsystem/3(a)
  Demux2/2 -> Subsystem1/3(a)
  Demux2/3 -> Subsystem2/3(a)
  Demux1/1 -> Subsystem/2(alpha)
  Demux1/2 -> Subsystem1/2(alpha)
  Demux1/3 -> Subsystem2/2(alpha)
  Degrees to Radians/1 -> Demux/1
  q[1..3]/1 -> Degrees to Radians/1, Display3/1
  Add/1 -> Subsystem1/1(q)
  Constant1/1 -> Add/2
  Demux/2 -> Add/1
  Constant3/1 -> Add1/2
  Demux/3 -> Add1/1
  Add1/1 -> Subsystem2/1(q)
  Demux/1 -> Add2/1
  Add2/1 -> Subsystem/1(q)
  Constant4/1 -> Add2/2

== system Simulatore_COMAU_UBHAND_IV_manipolazione_interna/SCOPES/Direct_kinematic2/Direct_kinematic_Comau/T_3_6 ==
  q[1..3] : Inport
  Add : Sum  Inputs=-+
  Add1 : Sum  Inputs=-+
  Add2 : Sum  Inputs=-+
  Constant : Constant  Value=[alpha_c(4) alpha_c(5) alpha_c(6) ]
  Constant1 : Constant  Value=teta_c(5)
  Constant2 : Constant  Value=[a_c(4) a_c(5) a_c(6)]
  Constant3 : Constant  Value=teta_c(6)
  Constant4 : Constant  Value=teta_c(4)
  Degrees to Radians : simulink_extras/Transformations/Degrees to Radians
  Demux : Demux  Outputs=3
  Demux1 : Demux  Outputs=3
  Demux2 : Demux  Outputs=3
  Display3 : Display
  Matrix Multiply : Product  Multiplication=Matrix(*)
  Matrix Multiply1 : Product  Multiplication=Matrix(*)
  Subsystem : Subsystem
  Subsystem1 : Subsystem
  Subsystem2 : Subsystem
  T_3_6 : Outport
  wires:
  q[1..3]/1 -> Display3/1, Degrees to Radians/1
  Degrees to Radians/1 -> Demux/1
  Demux1/3 -> Subsystem2/2(alpha)
  Demux1/2 -> Subsystem1/2(alpha)
  Demux1/1 -> Subsystem/2(alpha)
  Demux2/3 -> Subsystem2/3(a)
  Demux2/2 -> Subsystem1/3(a)
  Demux2/1 -> Subsystem/3(a)
  Constant2/1 -> Demux2/1
  Matrix Multiply1/1 -> T_3_6/1
  Subsystem2/1(Out1) -> Matrix Multiply1/2
  Matrix Multiply/1 -> Matrix Multiply1/1
  Subsystem1/1(Out1) -> Matrix Multiply/2
  Subsystem/1(Out1) -> Matrix Multiply/1
  Constant/1 -> Demux1/1
  Add/1 -> Subsystem1/1(q)
  Constant1/1 -> Add/2
  Demux/2 -> Add/1
  Constant3/1 -> Add1/2
  Demux/3 -> Add1/1
  Demux/1 -> Add2/1
  Add2/1 -> Subsystem/1(q)
  Constant4/1 -> Add2/2
  Add1/1 -> Subsystem2/1(q)

== system Simulatore_COMAU_UBHAND_IV_manipolazione_interna/SCOPES/Direct_kinematic2/Direct_kinematic_hand/Direct_kinematic_index ==
  Joints_reference : Inport  PortDimensions=3
  T_0f : Inport  Port=2
  Constant : Constant  Value=[alpha_f(2,1) alpha_f(2,2) alpha_f(2,3) alpha_f(2,4)]
  Constant2 : Constant  Value=[a_f(2,1) a_f(2,2) a_f(2,3) a_f(2,4)]
  Degrees to Radians : simulink_extras/Transformations/Degrees to Radians
  Demux : Demux
  Demux1 : Demux
  Demux2 : Demux
  Demux3 : Demux  Outputs=3
  Display3 : Display
  Gain : Gain  Gain=0.7
  Matrix Multiply{,1..3} (4 alike) : Product  Multiplication=Matrix(*)
  Subsystem : Subsystem
  Subsystem1 : Subsystem
  Subsystem2 : Subsystem
  Subsystem3 : Subsystem
  Vector Concatenate : Concatenate  Mode=Vector
  T_finger_tip : Outport
  wires:
  Degrees to Radians/1 -> Demux/1
  Vector Concatenate/1 -> Display3/1, Degrees to Radians/1
  Gain/1 -> Vector Concatenate/2
  Demux3/3 -> Gain/1
  Joints_reference/1 -> Vector Concatenate/1, Demux3/1
  Demux1/4 -> Subsystem3/2(alpha)
  Demux1/3 -> Subsystem2/2(alpha)
  Demux1/2 -> Subsystem1/2(alpha)
  Demux1/1 -> Subsystem/2(alpha)
  Demux2/4 -> Subsystem3/3(a)
  Demux2/3 -> Subsystem2/3(a)
  Demux2/2 -> Subsystem1/3(a)
  Demux2/1 -> Subsystem/3(a)
  Demux/4 -> Subsystem3/1(q)
  Demux/3 -> Subsystem2/1(q)
  Demux/2 -> Subsystem1/1(q)
  Demux/1 -> Subsystem/1(q)
  Constant2/1 -> Demux2/1
  Matrix Multiply2/1 -> T_finger_tip/1
  Matrix Multiply1/1 -> Matrix Multiply2/1
  Subsystem3/1(Out1) -> Matrix Multiply2/2
  Subsystem2/1(Out1) -> Matrix Multiply1/2
  Matrix Multiply/1 -> Matrix Multiply1/1
  Subsystem1/1(Out1) -> Matrix Multiply/2
  Subsystem/1(Out1) -> Matrix Multiply3/2
  Constant/1 -> Demux1/1
  T_0f/1 -> Matrix Multiply3/1
  Matrix Multiply3/1 -> Matrix Multiply/1

== system Simulatore_COMAU_UBHAND_IV_manipolazione_interna/SCOPES/Direct_kinematic2/Direct_kinematic_hand/Direct_kinematic_little ==
  Joints_reference : Inport  PortDimensions=3
  T_0f : Inport  Port=2
  Constant : Constant  Value=[alpha_f(5,1) alpha_f(5,2) alpha_f(5,3) alpha_f(5,4)]
  Constant2 : Constant  Value=[a_f(5,1) a_f(5,2) a_f(5,3) a_f(5,4)]
  Degrees to Radians : simulink_extras/Transformations/Degrees to Radians
  Demux : Demux
  Demux1 : Demux
  Demux2 : Demux
  Demux3 : Demux  Outputs=3
  Display3 : Display
  Gain : Gain  Gain=0.7
  Matrix Multiply{,1..3} (4 alike) : Product  Multiplication=Matrix(*)
  Subsystem : Subsystem
  Subsystem1 : Subsystem
  Subsystem2 : Subsystem
  Subsystem3 : Subsystem
  Vector Concatenate : Concatenate  Mode=Vector
  T_finger_tip : Outport
  wires:
  Degrees to Radians/1 -> Demux/1
  Vector Concatenate/1 -> Display3/1, Degrees to Radians/1
  Gain/1 -> Vector Concatenate/2
  Demux3/3 -> Gain/1
  Joints_reference/1 -> Vector Concatenate/1, Demux3/1
  Demux1/4 -> Subsystem3/2(alpha)
  Demux1/3 -> Subsystem2/2(alpha)
  Demux1/2 -> Subsystem1/2(alpha)
  Demux1/1 -> Subsystem/2(alpha)
  Demux2/4 -> Subsystem3/3(a)
  Demux2/3 -> Subsystem2/3(a)
  Demux2/2 -> Subsystem1/3(a)
  Demux2/1 -> Subsystem/3(a)
  Demux/4 -> Subsystem3/1(q)
  Demux/3 -> Subsystem2/1(q)
  Demux/2 -> Subsystem1/1(q)
  Demux/1 -> Subsystem/1(q)
  Constant2/1 -> Demux2/1
  Matrix Multiply2/1 -> T_finger_tip/1
  Matrix Multiply1/1 -> Matrix Multiply2/1
  Subsystem3/1(Out1) -> Matrix Multiply2/2
  Subsystem2/1(Out1) -> Matrix Multiply1/2
  Matrix Multiply/1 -> Matrix Multiply1/1
  Subsystem1/1(Out1) -> Matrix Multiply/2
  Subsystem/1(Out1) -> Matrix Multiply3/2
  Constant/1 -> Demux1/1
  T_0f/1 -> Matrix Multiply3/1
  Matrix Multiply3/1 -> Matrix Multiply/1

== system Simulatore_COMAU_UBHAND_IV_manipolazione_interna/SCOPES/Direct_kinematic2/Direct_kinematic_hand/Direct_kinematic_middle ==
  Joints_reference : Inport  PortDimensions=3
  T_0f : Inport  Port=2
  Constant : Constant  Value=[alpha_f(3,1) alpha_f(3,2) alpha_f(3,3) alpha_f(3,4)]
  Constant2 : Constant  Value=[a_f(3,1) a_f(3,2) a_f(3,3) a_f(3,4)]
  Degrees to Radians : simulink_extras/Transformations/Degrees to Radians
  Demux : Demux
  Demux1 : Demux
  Demux2 : Demux
  Demux3 : Demux  Outputs=3
  Display3 : Display
  Gain : Gain  Gain=0.7
  Matrix Multiply{,1..3} (4 alike) : Product  Multiplication=Matrix(*)
  Subsystem : Subsystem
  Subsystem1 : Subsystem
  Subsystem2 : Subsystem
  Subsystem3 : Subsystem
  Vector Concatenate : Concatenate  Mode=Vector
  T_finger_tip : Outport
  wires:
  Constant/1 -> Demux1/1
  Subsystem/1(Out1) -> Matrix Multiply3/2
  Subsystem1/1(Out1) -> Matrix Multiply/2
  Matrix Multiply/1 -> Matrix Multiply1/1
  Subsystem2/1(Out1) -> Matrix Multiply1/2
  Subsystem3/1(Out1) -> Matrix Multiply2/2
  Matrix Multiply1/1 -> Matrix Multiply2/1
  Matrix Multiply2/1 -> T_finger_tip/1
  Constant2/1 -> Demux2/1
  Demux/1 -> Subsystem/1(q)
  Demux/2 -> Subsystem1/1(q)
  Demux/3 -> Subsystem2/1(q)
  Demux/4 -> Subsystem3/1(q)
  Demux2/1 -> Subsystem/3(a)
  Demux2/2 -> Subsystem1/3(a)
  Demux2/3 -> Subsystem2/3(a)
  Demux2/4 -> Subsystem3/3(a)
  Demux1/1 -> Subsystem/2(alpha)
  Demux1/2 -> Subsystem1/2(alpha)
  Demux1/3 -> Subsystem2/2(alpha)
  Demux1/4 -> Subsystem3/2(alpha)
  Joints_reference/1 -> Demux3/1, Vector Concatenate/1
  Demux3/3 -> Gain/1
  Gain/1 -> Vector Concatenate/2
  Vector Concatenate/1 -> Degrees to Radians/1, Display3/1
  Degrees to Radians/1 -> Demux/1
  T_0f/1 -> Matrix Multiply3/1
  Matrix Multiply3/1 -> Matrix Multiply/1

== system Simulatore_COMAU_UBHAND_IV_manipolazione_interna/SCOPES/Direct_kinematic2/Direct_kinematic_hand/Direct_kinematic_ring ==
  Joints_reference : Inport  PortDimensions=3
  T_0f : Inport  Port=2
  Constant : Constant  Value=[alpha_f(4,1) alpha_f(4,2) alpha_f(4,3) alpha_f(4,4)]
  Constant2 : Constant  Value=[a_f(4,1) a_f(4,2) a_f(4,3) a_f(4,4)]
  Degrees to Radians : simulink_extras/Transformations/Degrees to Radians
  Demux : Demux
  Demux1 : Demux
  Demux2 : Demux
  Demux3 : Demux  Outputs=3
  Display3 : Display
  Gain : Gain  Gain=0.7
  Matrix Multiply{,1..3} (4 alike) : Product  Multiplication=Matrix(*)
  Subsystem : Subsystem
  Subsystem1 : Subsystem
  Subsystem2 : Subsystem
  Subsystem3 : Subsystem
  Vector Concatenate : Concatenate  Mode=Vector
  T_finger_tip : Outport
  wires:
  Degrees to Radians/1 -> Demux/1
  Vector Concatenate/1 -> Display3/1, Degrees to Radians/1
  Gain/1 -> Vector Concatenate/2
  Demux3/3 -> Gain/1
  Joints_reference/1 -> Vector Concatenate/1, Demux3/1
  Demux1/4 -> Subsystem3/2(alpha)
  Demux1/3 -> Subsystem2/2(alpha)
  Demux1/2 -> Subsystem1/2(alpha)
  Demux1/1 -> Subsystem/2(alpha)
  Demux2/4 -> Subsystem3/3(a)
  Demux2/3 -> Subsystem2/3(a)
  Demux2/2 -> Subsystem1/3(a)
  Demux2/1 -> Subsystem/3(a)
  Demux/4 -> Subsystem3/1(q)
  Demux/3 -> Subsystem2/1(q)
  Demux/2 -> Subsystem1/1(q)
  Demux/1 -> Subsystem/1(q)
  Constant2/1 -> Demux2/1
  Matrix Multiply2/1 -> T_finger_tip/1
  Matrix Multiply1/1 -> Matrix Multiply2/1
  Subsystem3/1(Out1) -> Matrix Multiply2/2
  Subsystem2/1(Out1) -> Matrix Multiply1/2
  Matrix Multiply/1 -> Matrix Multiply1/1
  Subsystem1/1(Out1) -> Matrix Multiply/2
  Subsystem/1(Out1) -> Matrix Multiply3/2
  Constant/1 -> Demux1/1
  T_0f/1 -> Matrix Multiply3/1
  Matrix Multiply3/1 -> Matrix Multiply/1
